simulink model "TosAeb"  (R2021a)
settings: Solver=FixedStepDiscrete  StartTime=0.0  StopTime=inf  FixedStep=0.01  RelTol=1e-3
undefined symbols (not defined in the file or in its folder): ACCModSts, AccSts1, AEB_ObjectMovingOrientation, AEB_ObjectMovingState, CN_BTCActive, CN_CurrentGearR, CN_CurrentGearR_DHT, CN_PTCActive, CN_VDCActive, CN_VSC_EspOff, CN_VSC_FCW_AEB_Error, CN_VSC_FCW_AEB_NoDispay, CN_VSC_FCW_AEB_PerfmDgdtn, CN_VSC_FCW_AEB_TempUnAvlbl, CN_VSC_VehStandstill, CNaccelAvoidMax, CNaccelAvoidMin, CNbrakePedalPress, CNcomfortStrLeftAccelMax, CNcomfortStrLeftAccelMin, CNcomfortStrRightAccelMax, CNcomfortStrRightAccelMin, CNDrvDoorOpen, CNDrvModeEco, CNDrvModeNorm, CNDrvModeOther, CNDrvModeSport, CNDrvSBRreminder, CNNull, CNobjInvalidID, CNPassDoorOpen, CNPassSBRreminder, CNPEBSAccelNegInf, CNPEBSAccelNull, CNPEBSTimeInf, CNPEBSTimeNull, CNSTOPMAX, CNSTOPNULL, CNsubjWidth, CNSwtOFF, CNSwtON, CNTTCMAX, CNTTCNULL, CNVehConfigAEBCycNone, CNVehConfigAEBNone, CNVehConfigAEBPedNone, CNVehConfigFCWNone, CNVehConfigJANone, CollisionSeverity, FALSE, FCWSnvty, JACycObjCalcInfoDefaultParams, JAPedObjCalcInfoDefaultParams, JAVehObjCalcInfoDefaultParams, latCycObjCalcInfoDefaultParams, latPedObjCalcInfoDefaultParams, longObjCalcInfoDefaultParams, ModSts, movingStatus, ObjectType ... (+1112)
libraries: simulink x3031, AEBSFunctionLib x50, Ta_Library x33, AEBSStateMachineLib x23, Mylibrary x12, simulink_extras x7, ObjSelect_lib x3, dspstat3 x2
(part 13 of 102)

== system TosAeb/AEB_Objsel/TA_Processor/AEB_ObjSelect/TargetSelect/Lat_ObjSelect/Lat_ValidNumber ==
  LatObj_Valid : Inport
  Add : Sum
  Compare To Constant : simulink/Logic and Bit Operations/Compare To Constant  relop===  const=1  OutDataTypeStr=boolean
  Compare To Constant1 : simulink/Logic and Bit Operations/Compare To Constant  relop=>  const=1  OutDataTypeStr=boolean
  Compare To Constant2 : simulink/Logic and Bit Operations/Compare To Constant  relop===  const=1  OutDataTypeStr=boolean
  Constant : Constant  Value=0  OutDataTypeStr=uint8
  Constant1 : Constant  OutDataTypeStr=uint8
  Constant2 : Constant  OutDataTypeStr=uint8
  For Iterator : ForIterator  IterationLimit=32
  Index Vector : MultiPortSwitch  Inputs=1
  Logical Operator : Logic  AllPortsSameDT=off  OutDataTypeStr=boolean
  Saturation1 : Saturate  UpperLimit=254  LowerLimit=0
  Switch : Switch  Criteria=u2 > Threshold
  Switch1 : Switch  Criteria=u2 > Threshold
  Switch2 : Switch  Criteria=u2 > Threshold
  Unit Delay : UnitDelay  SampleTime=-1  HasFrameUpgradeWarning=on
  Lat_ObjNum : Outport
  wires:
  LatObj_Valid/1 -> Index Vector/2
  For Iterator/1 -> [ID], Index Vector/1
  Index Vector/1 -> Compare To Constant/1
  Compare To Constant1/1 -> Switch/2
  Constant/1 -> Switch2/3
  Compare To Constant/1 -> Switch1/2, Logical Operator/1
  Add/1 -> Switch1/1
  Constant1/1 -> Add/2
  Unit Delay/1 -> Add/1, Switch1/3
  [ID] -> Compare To Constant1/1
  Switch1/1 -> Switch/1
  Switch/1 -> Saturation1/1
  Saturation1/1 -> Lat_ObjNum/1, Unit Delay/1
  Compare To Constant2/1 -> Logical Operator/2
  [ID] -> Compare To Constant2/1
  Logical Operator/1 -> Switch2/2
  Constant2/1 -> Switch2/1
  Switch2/1 -> Switch/3

== system TosAeb/AEB_Objsel/TA_Processor/AEB_ObjSelect/TargetSelect/Long_ObjSelect/Obj_IDConfirm ==
  TA_ObjInfo : Inport
  VehSelfData : Inport  Port=2
  Boudingbox_Calculate : Inport  Port=3
  Obj_Coef : Inport  Port=4
  TTI : Inport  Port=5
  AccTgtIdn : Inport  Port=6
  Overlap_Rate : Inport  Port=7
  Robust : Subsystem
  Target_Select_decle : Subsystem
  ValidTarget_ID : Outport
  ID_num : Outport  Port=2
  long_TTC : Outport  Port=3
  wires:
  Target_Select_decle/1(ValidTarget_ID) -> Robust/1(ValidTarget_ID)
  Target_Select_decle/2(Num) -> Robust/2(Num_IN)
  Target_Select_decle/3(long_TTC) -> long_TTC/1
  Overlap_Rate/1 -> Target_Select_decle/7(Overlap_Rate)
  AccTgtIdn/1 -> Target_Select_decle/6(AccTgtIdn)
  Boudingbox_Calculate/1 -> Target_Select_decle/3(Boudingbox_Calculate)
  TA_ObjInfo/1 -> Target_Select_decle/1(TA_ObjInfo)
  TTI/1 -> Target_Select_decle/5(TTI)
  Obj_Coef/1 -> Target_Select_decle/4(Obj_Coef)
  Robust/1(ID) -> ValidTarget_ID/1
  VehSelfData/1 -> Target_Select_decle/2(VehSelfData)
  Robust/2(Num) -> ID_num/1

== system TosAeb/AEB_Objsel/TA_Processor/PreProcessor/ObjectPrecessor/TAObjInfoCalc/For Each Subsystem ==
  Cam_ObsID_u8 : Inport
  Cam_ObsValid_u8 : Inport  Port=2
  Cam_ObsAge_u16 : Inport  Port=3
  Cam_ObsConfidence_u8 : Inport  Port=4
  Cam_ObsMotionCategory_u8 : Inport  Port=5
  Cam_ObsMotionStatus_u8 : Inport  Port=6
  Cam_ObsMeasureStatus_u8 : Inport  Port=7
  Cam_ObsClass_u8 : Inport  Port=8
  Cam_ObsVhSubType_u8 : Inport  Port=9
  Cam_ObsPedSubType_u8 : Inport  Port=10
  Cam_ObsPedPos_u8 : Inport  Port=11
  Cam_ObsPedOrientation_u8 : Inport  Port=12
  Cam_ObsLaneAssign_u8 : Inport  Port=13
  Cam_ObsLength_s : Inport  Port=14
  Cam_ObsWidth_s : Inport  Port=15
  Cam_ObsHeight_s : Inport  Port=16
  Cam_ObsCIPV_b : Inport  Port=17
  Cam_ObsMCP_b : Inport  Port=18
  Cam_ObsBlinker_u8 : Inport  Port=19
  Cam_ObsBrkLgt_b : Inport  Port=20
  Cam_ObsCenterAngle_s : Inport  Port=21
  Cam_ObsPosType_u8 : Inport  Port=22
  Cam_ObsPosX_s : Inport  Port=23
  Cam_ObsPosY_s : Inport  Port=24
  Cam_ObsVelX_s : Inport  Port=25
  Cam_ObsVelY_s : Inport  Port=26
  Cam_ObsAccX_s : Inport  Port=27
  Cam_ObsPosXSTD_s : Inport  Port=28
  Cam_ObsPosYSTD_s : Inport  Port=29
  Cam_ObsVelXSTD_s : Inport  Port=30
  Cam_ObsVelYSTD_s : Inport  Port=31
  Cam_ObsAccY_s : Inport  Port=32
  Cam_ObsAngle_s : Inport  Port=33
  Cam_ObsAngleSTD_s : Inport  Port=34
  Cam_ObsVelXAbs_s : Inport  Port=35
  Cam_ObsVelYAbs_s : Inport  Port=36
  Cam_ObsCornerPntX_s : Inport  Port=37
  Cam_ObsCutInDis_s : Inport  Port=38
  Cam_ObsCornerPntY_s : Inport  Port=39
  Cam_ObsCutInFlg_u8 : Inport  Port=40
  Cam_ObsCutInSpd_s : Inport  Port=41
  Cam_ObsCutInSpdValid_b : Inport  Port=42
  Cam_ObsProNotOnRoad_b : Inport  Port=43
  Cam_ObsProCoverdByOthers_b : Inport  Port=44
  Cam_ObsProObserveJump_b : Inport  Port=45
  Cam_ObsProObserveSide_b : Inport  Port=46
  Cam_ObsProBtTapLevel1_b : Inport  Port=47
  Cam_ObsProBtTapLevel2_b : Inport  Port=48
  Cam_ObsProBtTapLevel3_b : Inport  Port=49
  IDForEach : Inport  Port=50  Partition=on  PartitionDimension=1  PartitionWidth=1  PartitionOffset=0
  VehSelfIn : Inport  Port=51
  For Each : ForEach  DisableCoverage=on  ShowIterationIndex=on
  ForEachInput : Subsystem
  Info_Check1 : Subsystem
  Terminator : Terminator
  Fus_ObjID : Outport  ConcatenationDimension=1
  Fus_ObjOrientation : Outport  Port=2  ConcatenationDimension=1
  Fus_ObjState : Outport  Port=3  ConcatenationDimension=1
  Fus_ObjfusType : Outport  Port=4  ConcatenationDimension=1
  Fus_ObjLaneAssign : Outport  Port=5  ConcatenationDimension=1
  Fus_ObjDy : Outport  Port=6  ConcatenationDimension=1
  Fus_ObjDx : Outport  Port=7  ConcatenationDimension=1
  Fus_ObjVx : Outport  Port=8  ConcatenationDimension=1
  Fus_ObjVy : Outport  Port=9  ConcatenationDimension=1
  Fus_ObjQuality : Outport  Port=10  ConcatenationDimension=1
  Fus_ObjAy : Outport  Port=11  ConcatenationDimension=1
  Fus_ObjAx : Outport  Port=12  ConcatenationDimension=1
  Fus_ObjVxAbs : Outport  Port=13  ConcatenationDimension=1
  Fus_ObjVyAbs : Outport  Port=14  ConcatenationDimension=1
  Fus_ObjHeadAngle : Outport  Port=15  ConcatenationDimension=1
  Fus_ObjType : Outport  Port=16  ConcatenationDimension=1
  Fus_ObjLength : Outport  Port=17  ConcatenationDimension=1
  Fus_ObjWidth : Outport  Port=18  ConcatenationDimension=1
  Fus_ObjHight : Outport  Port=19  ConcatenationDimension=1
  Fus_dxSTD : Outport  Port=20  ConcatenationDimension=1
  Fus_dySTD : Outport  Port=21  ConcatenationDimension=1
  Fus_VelxSTD : Outport  Port=22  ConcatenationDimension=1
  Fus_VelySTD : Outport  Port=23  ConcatenationDimension=1
  Fus_HeadAngleSTD : Outport  Port=24  ConcatenationDimension=1
  Fus_CenterAngle : Outport  Port=25  ConcatenationDimension=1
  Fus_AxAbs : Outport  Port=26  ConcatenationDimension=1
  Fus_AyAbs : Outport  Port=27  ConcatenationDimension=1
  wires:
  VehSelfIn/1 -> Info_Check1/2(VehSelfIn)
  Info_Check1/1(Fus_ObjID) -> Fus_ObjID/1
  Info_Check1/2(Fus_ObjOrientation) -> Fus_ObjOrientation/1
  Info_Check1/3(Fus_ObjState) -> Fus_ObjState/1
  Info_Check1/4(Fus_ObjfusType) -> Fus_ObjfusType/1
  Info_Check1/5(Fus_ObjLaneAssign) -> Fus_ObjLaneAssign/1
  Info_Check1/6(Fus_ObjDy) -> Fus_ObjDy/1
  Info_Check1/7(Fus_ObjDx) -> Fus_ObjDx/1
  Info_Check1/8(Fus_ObjVx) -> Fus_ObjVx/1
  Info_Check1/9(Fus_ObjVy) -> Fus_ObjVy/1
  Info_Check1/10(Fus_ObjQuality) -> Fus_ObjQuality/1
  Info_Check1/11(Fus_ObjAy) -> Fus_ObjAy/1
  Info_Check1/12(Fus_ObjAx) -> Fus_ObjAx/1
  Info_Check1/13(Fus_ObjVxAbs) -> Fus_ObjVxAbs/1
  Info_Check1/14(Fus_ObjVyAbs) -> Fus_ObjVyAbs/1
  Info_Check1/15(Fus_ObjHeadAngle) -> Fus_ObjHeadAngle/1
  Info_Check1/16(Fus_ObjType) -> Fus_ObjType/1
  Info_Check1/17(Fus_ObjLength) -> Fus_ObjLength/1
  Info_Check1/18(Fus_ObjWidth) -> Fus_ObjWidth/1
  Info_Check1/19(Fus_ObjHight) -> Fus_ObjHight/1
  Info_Check1/20(Fus_dxSTD) -> Fus_dxSTD/1
  Info_Check1/21(Fus_dySTD) -> Fus_dySTD/1
  Info_Check1/22(Fus_VelxSTD) -> Fus_VelxSTD/1
  Info_Check1/23(Fus_VelySTD) -> Fus_VelySTD/1
  Info_Check1/24(Fus_HeadAngleSTD) -> Fus_HeadAngleSTD/1
  Info_Check1/25(Fus_CenterAngle) -> Fus_CenterAngle/1
  Info_Check1/26(Fus_AxAbs) -> Fus_AxAbs/1
  Info_Check1/27(Fus_AyAbs) -> Fus_AyAbs/1
  ForEachInput/1(Obs_Infomation) -> Info_Check1/1(Obs_Infomation)
  Cam_ObsID_u8/1 -> ForEachInput/2(Cam_ObsID_u8)
  Cam_ObsValid_u8/1 -> ForEachInput/3(Cam_ObsValid_u8)
  Cam_ObsAge_u16/1 -> ForEachInput/4(Cam_ObsAge_u16)
  Cam_ObsConfidence_u8/1 -> ForEachInput/5(Cam_ObsConfidence_u8)
  Cam_ObsMotionCategory_u8/1 -> ForEachInput/6(Cam_ObsMotionCategory_u8)
  Cam_ObsMotionStatus_u8/1 -> ForEachInput/7(Cam_ObsMotionStatus_u8)
  Cam_ObsMeasureStatus_u8/1 -> ForEachInput/8(Cam_ObsMeasureStatus_u8)
  Cam_ObsClass_u8/1 -> ForEachInput/9(Cam_ObsClass_u8)
  Cam_ObsVhSubType_u8/1 -> ForEachInput/10(Cam_ObsVhSubType_u8)
  Cam_ObsPedSubType_u8/1 -> ForEachInput/11(Cam_ObsPedSubType_u8)
  Cam_ObsPedPos_u8/1 -> ForEachInput/12(Cam_ObsPedPos_u8)
  Cam_ObsPedOrientation_u8/1 -> ForEachInput/13(Cam_ObsPedOrientation_u8)
  Cam_ObsLaneAssign_u8/1 -> ForEachInput/14(Cam_ObsLaneAssign_u8)
  Cam_ObsLength_s/1 -> ForEachInput/15(Cam_ObsLength_s)
  Cam_ObsWidth_s/1 -> ForEachInput/16(Cam_ObsWidth_s)
  Cam_ObsHeight_s/1 -> ForEachInput/17(Cam_ObsHeight_s)
  Cam_ObsCIPV_b/1 -> ForEachInput/18(Cam_ObsCIPV_b)
  Cam_ObsMCP_b/1 -> ForEachInput/19(Cam_ObsMCP_b)
  Cam_ObsBlinker_u8/1 -> ForEachInput/20(Cam_ObsBlinker_u8)
  Cam_ObsBrkLgt_b/1 -> ForEachInput/21(Cam_ObsBrkLgt_b)
  Cam_ObsCenterAngle_s/1 -> ForEachInput/22(Cam_ObsCenterAngle_s)
  Cam_ObsPosType_u8/1 -> ForEachInput/23(Cam_ObsPosType_u8)
  Cam_ObsPosX_s/1 -> ForEachInput/24(Cam_ObsPosX_s)
  Cam_ObsPosY_s/1 -> ForEachInput/25(Cam_ObsPosY_s)
  Cam_ObsVelX_s/1 -> ForEachInput/26(Cam_ObsVelX_s)
  Cam_ObsVelY_s/1 -> ForEachInput/27(Cam_ObsVelY_s)
  Cam_ObsAccX_s/1 -> ForEachInput/28(Cam_ObsAccX_s)
  Cam_ObsPosXSTD_s/1 -> ForEachInput/29(Cam_ObsPosXSTD_s)
  Cam_ObsPosYSTD_s/1 -> ForEachInput/30(Cam_ObsPosYSTD_s)
  Cam_ObsVelXSTD_s/1 -> ForEachInput/31(Cam_ObsVelXSTD_s)
  Cam_ObsVelYSTD_s/1 -> ForEachInput/32(Cam_ObsVelYSTD_s)
  Cam_ObsAccY_s/1 -> ForEachInput/33(Cam_ObsAccY_s)
  Cam_ObsAngle_s/1 -> ForEachInput/34(Cam_ObsAngle_s)
  Cam_ObsAngleSTD_s/1 -> ForEachInput/35(Cam_ObsAngleSTD_s)
  Cam_ObsVelXAbs_s/1 -> ForEachInput/36(Cam_ObsVelXAbs_s)
  Cam_ObsVelYAbs_s/1 -> ForEachInput/37(Cam_ObsVelYAbs_s)
  Cam_ObsCornerPntX_s/1 -> ForEachInput/38(Cam_ObsCornerPntX_s)
  Cam_ObsCutInDis_s/1 -> ForEachInput/39(Cam_ObsCutInDis_s)
  Cam_ObsCornerPntY_s/1 -> ForEachInput/40(Cam_ObsCornerPntY_s)
  Cam_ObsCutInFlg_u8/1 -> ForEachInput/41(Cam_ObsCutInFlg_u8)
  Cam_ObsCutInSpd_s/1 -> ForEachInput/42(Cam_ObsCutInSpd_s)
  Cam_ObsCutInSpdValid_b/1 -> ForEachInput/43(Cam_ObsCutInSpdValid_b)
  Cam_ObsProNotOnRoad_b/1 -> ForEachInput/44(Cam_ObsProNotOnRoad_b)
  Cam_ObsProCoverdByOthers_b/1 -> ForEachInput/45(Cam_ObsProCoverdByOthers_b)
  Cam_ObsProObserveJump_b/1 -> ForEachInput/46(Cam_ObsProObserveJump_b)
  Cam_ObsProObserveSide_b/1 -> ForEachInput/47(Cam_ObsProObserveSide_b)
  Cam_ObsProBtTapLevel1_b/1 -> ForEachInput/48(Cam_ObsProBtTapLevel1_b)
  Cam_ObsProBtTapLevel2_b/1 -> ForEachInput/49(Cam_ObsProBtTapLevel2_b)
  Cam_ObsProBtTapLevel3_b/1 -> ForEachInput/50(Cam_ObsProBtTapLevel3_b)
  IDForEach/1 -> Terminator/1
  For Each/1 -> ForEachInput/1(Index)

== system TosAeb/AEB_Objsel/TA_Processor/PreProcessor/ObjectPrecessor/TAObjInfoCalc/Signal_Assignment ==
  Fus_ObjID : Inport
  Fus_ObjOrientation : Inport  Port=2
  Fus_ObjState : Inport  Port=3
  Fus_ObjfusType : Inport  Port=4
  Fus_ObjLaneAssign : Inport  Port=5
  Fus_ObjDy : Inport  Port=6
  Fus_ObjDx : Inport  Port=7
  Fus_ObjVx : Inport  Port=8
  Fus_ObjVy : Inport  Port=9
  Fus_ObjQuality : Inport  Port=10
  Fus_ObjAy : Inport  Port=11
  Fus_ObjAx : Inport  Port=12
  Fus_ObjVxAbs : Inport  Port=13
  Fus_ObjVyAbs : Inport  Port=14
  Fus_ObjHeadAngle : Inport  Port=15
  Fus_ObjType : Inport  Port=16
  Fus_ObjLength : Inport  Port=17
  Fus_ObjWidth : Inport  Port=18
  Fus_ObjHight : Inport  Port=19
  Fus_dxSTD : Inport  Port=20
  Fus_dySTD : Inport  Port=21
  Fus_VelxSTD : Inport  Port=22
  Fus_VelySTD : Inport  Port=23
  Fus_HeadAngleSTD : Inport  Port=24
  Fus_CenterAngle : Inport  Port=25
  Fus_AxAbs : Inport  Port=26
  Fus_AyAbs : Inport  Port=27
  Bus Creator : BusCreator  Inputs=27  OutDataTypeStr=Bus: TA_ObjInfo  NonVirtualBus=on
  Signal Conversion{,1..26} (27 alike) : SignalConversion  OverrideOpt=off
  TA_ObjInfo : Outport
  wires:
  Fus_ObjID/1 -> Signal Conversion/1
  Fus_ObjState/1 -> Signal Conversion2/1
  Fus_ObjOrientation/1 -> Signal Conversion3/1
  Fus_ObjfusType/1 -> Signal Conversion4/1
  Fus_ObjType/1 -> Signal Conversion1/1
  Fus_ObjLaneAssign/1 -> Signal Conversion5/1
  Fus_ObjDx/1 -> Signal Conversion6/1
  Fus_ObjDy/1 -> Signal Conversion7/1
  Fus_dxSTD/1 -> Signal Conversion8/1
  Fus_dySTD/1 -> Signal Conversion9/1
  Fus_ObjVx/1 -> Signal Conversion10/1
  Fus_ObjVy/1 -> Signal Conversion11/1
  Fus_VelxSTD/1 -> Signal Conversion12/1
  Fus_VelySTD/1 -> Signal Conversion13/1
  Fus_ObjVxAbs/1 -> Signal Conversion14/1
  Fus_ObjVyAbs/1 -> Signal Conversion15/1
  Fus_ObjLength/1 -> Signal Conversion16/1
  Fus_ObjWidth/1 -> Signal Conversion17/1
  Fus_ObjHight/1 -> Signal Conversion18/1
  Fus_ObjAy/1 -> Signal Conversion20/1
  Fus_ObjAx/1 -> Signal Conversion19/1
  Fus_AxAbs/1 -> Signal Conversion21/1
  Fus_AyAbs/1 -> Signal Conversion22/1
  Fus_ObjQuality/1 -> Signal Conversion23/1
  Fus_ObjHeadAngle/1 -> Signal Conversion25/1
  Fus_HeadAngleSTD/1 -> Signal Conversion26/1
  Fus_CenterAngle/1 -> Signal Conversion24/1
  Signal Conversion21/1 -> Bus Creator/22  "TA_ObjAxAbs"
  Signal Conversion22/1 -> Bus Creator/23  "TA_ObjAyAbs"
  Signal Conversion20/1 -> Bus Creator/21  "TA_ObjAy"
  Signal Conversion23/1 -> Bus Creator/24  "TA_ObjConfidence"
  Signal Conversion19/1 -> Bus Creator/20  "TA_ObjAx"
  Signal Conversion24/1 -> Bus Creator/25  "TA_ObjCenterAngle"
  Signal Conversion18/1 -> Bus Creator/19  "TA_ObjHight"
  Signal Conversion17/1 -> Bus Creator/18  "TA_ObjWidth"
  Signal Conversion25/1 -> Bus Creator/26  "TA_ObjHeadAngle"
  Signal Conversion16/1 -> Bus Creator/17  "TA_ObjLength"
  Signal Conversion26/1 -> Bus Creator/27  "TA_ObjHeadAngleStd"
  Signal Conversion15/1 -> Bus Creator/16  "TA_ObjVyAbs"
  Signal Conversion14/1 -> Bus Creator/15  "TA_ObjVxAbs"
  Signal Conversion13/1 -> Bus Creator/14  "TA_ObjVyStd"
  Signal Conversion12/1 -> Bus Creator/13  "TA_ObjVxStd"
  Signal Conversion11/1 -> Bus Creator/12  "TA_ObjVy"
  Signal Conversion10/1 -> Bus Creator/11  "TA_ObjVx"
  Signal Conversion9/1 -> Bus Creator/10  "TA_ObjDyStd"
  Signal Conversion8/1 -> Bus Creator/9  "TA_ObjDxStd"
  Signal Conversion7/1 -> Bus Creator/8  "TA_ObjDy"
  Signal Conversion6/1 -> Bus Creator/7  "TA_ObjDx"
  Signal Conversion5/1 -> Bus Creator/6  "TA_ObjLaneAssign"
  Signal Conversion4/1 -> Bus Creator/5  "TA_ObjfusType"
  Signal Conversion3/1 -> Bus Creator/4  "TA_ObjOrientation"
  Signal Conversion2/1 -> Bus Creator/3  "TA_ObjState"
  Signal Conversion1/1 -> Bus Creator/2  "TA_ObjType"
  Signal Conversion/1 -> Bus Creator/1  "TA_ObjID"
  Bus Creator/1 -> TA_ObjInfo/1

== system TosAeb/AEB_Objsel/TA_Processor/PreProcessor/VehiclePreProcessor/CalculateCurvature/LowPassFilter ==
  note: Filter constant in low pass filter for pinion angle rate.
  note: 0.2
  PinionAngleRate_in : Inport
  Constant1 : Constant  Value=P_TA_PinionAgRateFilConInAsyEvlrCritEve
  LP1_1 : Subsystem
  PinionAngleRate : Outport
  wires:
  PinionAngleRate_in/1 -> LP1_1/1(U)
  Constant1/1 -> LP1_1/2(TsOverTau)
  LP1_1/1(Y) -> PinionAngleRate/1  "PinionAngleRate"

== system TosAeb/AEB_Objsel/TA_Processor/PreProcessor/VehiclePreProcessor/CalculateCurvature/SafeDivide ==
  Numerator : Inport  OutDataTypeStr=DataType
      settings: AttributesFormatString=DT=%<OutDataTypeStr>
  Denominator : Inport  Port=2  OutDataTypeStr=DataType
      settings: AttributesFormatString=DT=%<OutDataTypeStr>
  SafeDivide : MATLAB Function
  Quotient : Outport  OutDataTypeStr=DataType
      settings: AttributesFormatString=DT=%<OutDataTypeStr>
  wires:
  SafeDivide/1(Quotient) -> Quotient/1
  Denominator/1 -> SafeDivide/2(Denominator)  "Denominator"
  Numerator/1 -> SafeDivide/1(Numerator)  "Numerator"

== system TosAeb/AEB_Objsel/TA_Processor/PreProcessor/VehiclePreProcessor/CalculateCurvature/SafeDivide1 ==
  Numerator : Inport  OutDataTypeStr=DataType
      settings: AttributesFormatString=DT=%<OutDataTypeStr>
  Denominator : Inport  Port=2  OutDataTypeStr=DataType
      settings: AttributesFormatString=DT=%<OutDataTypeStr>
  SafeDivide : MATLAB Function
  Quotient : Outport  OutDataTypeStr=DataType
      settings: AttributesFormatString=DT=%<OutDataTypeStr>
  wires:
  SafeDivide/1(Quotient) -> Quotient/1
  Denominator/1 -> SafeDivide/2(Denominator)  "Denominator"
  Numerator/1 -> SafeDivide/1(Numerator)  "Numerator"

== system TosAeb/AEB_Objsel/TA_Processor/PreProcessor/VehiclePreProcessor/CalculateCurvature/StaticBicycleModelC0 ==
  note: 注：本模块使用方向盘角度作为输入
  VLgt : Inport
  PinionSteerAg : Inport  Port=2
  CalculateCurvatureCoG : Subsystem
  CalculateCurvatureRear : Subsystem
  CalculateDistanceToAxles : Subsystem
  CalculateSlip : Subsystem
  CalculateWheelAngle : Subsystem
  IntermediateCalculation : Subsystem
  CurvatureRear : Outport
  wires:
  CalculateCurvatureCoG/2(CurvatureCoG) -> CalculateSlip/1(CurvatureCoG)
  CalculateDistanceToAxles/1(DistCoGToRearAxle) -> [DistRe], CalculateCurvatureCoG/2(DistCoGToRearAxle)
  CalculateWheelAngle/1(WheelAngle) -> CalculateCurvatureCoG/1(WheelAngle)
  CalculateCurvatureCoG/1(RadiusCoG) -> CalculateCurvatureRear/1(RadiusCOG)
  VLgt/1 -> IntermediateCalculation/1(Speed)
  CalculateCurvatureRear/1(CurvatureRear) -> CurvatureRear/1
  PinionSteerAg/1 -> CalculateWheelAngle/1(PinionSteerAg)
  CalculateDistanceToAxles/2(DistCoGToFrontAxle) -> [DistFront], CalculateCurvatureCoG/3(DistCoGToFrontAxle)
  IntermediateCalculation/1(MassSpeedSquaredDivWheelBase) -> CalculateSlip/4(MassSpeedSquaredDivWheelBase), CalculateCurvatureCoG/4(MassSpeedSquaredDivWheelBase)
  [DistRe] -> CalculateSlip/2(DistCoGToRearAxle)
  [DistFront] -> CalculateSlip/3(DistCoGToFrontAxle)
  [DistRe] -> CalculateCurvatureRear/2(DistCoGToRearAxle)
  CalculateSlip/1(SlipAngle) -> CalculateCurvatureRear/3(SlipAngle)

== system TosAeb/AEB_Objsel/TA_Processor/PreProcessor/VehiclePreProcessor/CalculateCurvature/StaticBicycleModelC1 ==
  note: 注：本模块使用方向盘角速率作为输入
  VLgt : Inport
  PinionSteerAgRate : Inport  Port=2
  CalculateCurvatureCoG : Subsystem
  CalculateCurvatureRear : Subsystem
  CalculateDistanceToAxles : Subsystem
  CalculateSlip : Subsystem
  CalculateWheelAngle : Subsystem
  IntermediateCalculation : Subsystem
  CurvatureRear : Outport
  wires:
  CalculateWheelAngle/1(WheelAngle) -> CalculateCurvatureCoG/1(WheelAngle)
  CalculateCurvatureCoG/2(CurvatureCoG) -> CalculateSlip/1(CurvatureCoG)
  CalculateDistanceToAxles/1(DistCoGToRearAxle) -> [DistRe], CalculateCurvatureCoG/2(DistCoGToRearAxle)
  [DistRe] -> CalculateCurvatureRear/2(DistCoGToRearAxle)
  CalculateSlip/1(SlipAngle) -> CalculateCurvatureRear/3(SlipAngle)
  [DistFront] -> CalculateSlip/3(DistCoGToFrontAxle)
  VLgt/1 -> IntermediateCalculation/1(Speed)
  CalculateCurvatureRear/1(CurvatureRear) -> CurvatureRear/1
  CalculateCurvatureCoG/1(RadiusCoG) -> CalculateCurvatureRear/1(RadiusCOG)
  IntermediateCalculation/1(MassSpeedSquaredDivWheelBase) -> CalculateSlip/4(MassSpeedSquaredDivWheelBase), CalculateCurvatureCoG/4(MassSpeedSquaredDivWheelBase)
  [DistRe] -> CalculateSlip/2(DistCoGToRearAxle)
  CalculateDistanceToAxles/2(DistCoGToFrontAxle) -> [DistFront], CalculateCurvatureCoG/3(DistCoGToFrontAxle)
  PinionSteerAgRate/1 -> CalculateWheelAngle/1(PinionSteerAgRate)

== system TosAeb/AEB_Objsel/TA_Processor/PreProcessor/VehiclePreProcessor/CalculateCurvature/Weighting ==
  X1 : Inport
  WeightFactor : Inport  Port=2
  X2 : Inport  Port=3
  Add : Sum  Description=_3p3p4p5.ACC.000_11_  Inputs=-+
  Add1 : Sum  Description=_3p3p4p5.ACC.000_12_
  Constant : Constant  Description=_3p3p4p5.ACC.000_13_  Value=single(1)
  Product : Product  Description=_3p3p4p5.ACC.000_19_  RndMeth=Zero
  Product1 : Product  Description=_3p3p4p5.ACC.000_20_  RndMeth=Zero
  Saturation : Saturate  Description=_3p3p4p5.ACC.000_21_  UpperLimit=1  LowerLimit=0
  Y : Outport
  wires:
  WeightFactor/1 -> Saturation/1
  Saturation/1 -> Add/1, Product/2
  Add1/1 -> Y/1
  Product1/1 -> Add1/2
  Add/1 -> Product1/1
  X2/1 -> Product1/2
  Constant/1 -> Add/2
  Product/1 -> Add1/1
  X1/1 -> Product/1

== system TosAeb/AEB_Objsel/TA_Processor/PreProcessor/VehiclePreProcessor/CalculateCurvature/Weighting1 ==
  X1 : Inport
  WeightFactor : Inport  Port=2
  X2 : Inport  Port=3
  Add : Sum  Description=_3p3p4p5.ACC.000_11_  Inputs=-+
  Add1 : Sum  Description=_3p3p4p5.ACC.000_12_
  Constant : Constant  Description=_3p3p4p5.ACC.000_13_  Value=single(1)
  Product : Product  Description=_3p3p4p5.ACC.000_19_  RndMeth=Zero
  Product1 : Product  Description=_3p3p4p5.ACC.000_20_  RndMeth=Zero
  Saturation : Saturate  Description=_3p3p4p5.ACC.000_21_  UpperLimit=1  LowerLimit=0
  Y : Outport
  wires:
  WeightFactor/1 -> Saturation/1
  Saturation/1 -> Add/1, Product/2
  Add1/1 -> Y/1
  Product1/1 -> Add1/2
  Add/1 -> Product1/1
  X2/1 -> Product1/2
  Constant/1 -> Add/2
  Product/1 -> Add1/1
  X1/1 -> Product/1

== system TosAeb/AEB_Objsel/TA_Processor/PreProcessor/VehiclePreProcessor/PathEstimn_Calculate/For Iterator PahEstimn ==
  Curvature : Inport
  VehSelf : Inport  Port=2
  CurvatureRate : Inport  Port=3
  CheckVehicleStopping : Subsystem
  ComputeVehicleStateAtT1 : Subsystem
  For Iterator : ForIterator  IterationLimit=4
  InitialControlPointsCalculation : Subsystem
  PahEstimn1 : Subsystem
  SetVehicleStateAtT0 : Subsystem
  PahEstimn : Outport
  wires:
  For Iterator/1 -> [Index]
  [VehicleMotionT1] -> SetVehicleStateAtT0/5(VehicleMotionT1)  "VehicleMotionT1"
  [Index] -> SetVehicleStateAtT0/4(Index)  "Index"
  VehSelf/1 -> SetVehicleStateAtT0/1(VehSelf)
  SetVehicleStateAtT0/1(VehicleMotionT0) -> InitialControlPointsCalculation/2(VehicleMotionT0), CheckVehicleStopping/1(VehicleMotionT0), ComputeVehicleStateAtT1/1(VehicleMotionT0)  "VehicleMotionT0"
  CheckVehicleStopping/1(StoppingDistances) -> ComputeVehicleStateAtT1/2(StoppingDistances), InitialControlPointsCalculation/1(StoppingDistances)  "StoppingDistances"
  InitialControlPointsCalculation/1(ControlPoints) -> ComputeVehicleStateAtT1/3(ControlPoints), PahEstimn1/1(ControlPoints)
  ComputeVehicleStateAtT1/1(VehicleMotionT1) -> [VehicleMotionT1]
  [Index] -> PahEstimn1/2(Index)  "Index"
  PahEstimn1/1(PahEstimn) -> PahEstimn/1
  Curvature/1 -> SetVehicleStateAtT0/2(Curvature)
  CurvatureRate/1 -> SetVehicleStateAtT0/3(CurvatureRate)

== system TosAeb/ELK_Objsel/Target_Selection/If Action Subsystem/ELK_TargetSelection/If Action Threat_Assessment_Left/Delay_Trig ==
  Input : Inport
  Delay_Cnt : Inport  Port=2
  Chart : Stateflow chart  TreatAsAtomicUnit=on
  Output : Outport
  wires:
  Chart/1(Delay_Trig) -> Output/1
  Delay_Cnt/1 -> Chart/2(Delay_Trig_count)
  Input/1 -> Chart/1(Input)

== system TosAeb/ELK_Objsel/Target_Selection/If Action Subsystem/ELK_TargetSelection/If Action Threat_Assessment_Left/Delay_Trig1 ==
  Input : Inport
  Delay_Cnt : Inport  Port=2
  Chart : Stateflow chart  TreatAsAtomicUnit=on
  Output : Outport
  wires:
  Input/1 -> Chart/1(Input)
  Delay_Cnt/1 -> Chart/2(Delay_Trig_count)
  Chart/1(Delay_Trig) -> Output/1

== system TosAeb/ELK_Objsel/Target_Selection/If Action Subsystem/ELK_TargetSelection/If Action Threat_Assessment_Left/First_Order_Lag_Filter ==
  filt_loop_time_msec : Inport
  filt_tau_msec : Inport  Port=2
  unfiltered_input : Inport  Port=3
  Product2 : Product  Description=_GWMAB30P4.LKAFN.000_153_  Inputs=**  InputSameDT=on  OutDataTypeStr=Inherit: Same as first input
  Saturation : Saturate  Description=_GWMAB30P4.LKAFN.000_154_  UpperLimit=65535  LowerLimit=-65535
  Subsystem : Subsystem
  Sum : Sum  Description=_GWMAB30P4.LKAFN.000_155_  Inputs=+-  InputSameDT=on  OutDataTypeStr=Inherit: Same as first input
  Sum1 : Sum  Description=_GWMAB30P4.LKAFN.000_156_  InputSameDT=on  OutDataTypeStr=Inherit: Same as first input
  Unit Delay : UnitDelay  Description=_GWMAB30P4.LKAFN.000_157_  SampleTime=-1  HasFrameUpgradeWarning=on
  filtered_output : Outport
  wires:
  Subsystem/1(Gain) -> Product2/1
  filt_loop_time_msec/1 -> Subsystem/1(CL_TIME)
  Sum/1 -> Product2/2
  filt_tau_msec/1 -> Subsystem/2(time_constant)
  Sum1/1 -> Saturation/1
  unfiltered_input/1 -> Sum/1
  Product2/1 -> Sum1/1
  Saturation/1 -> filtered_output/1, Unit Delay/1
  Unit Delay/1 -> Sum/2, Sum1/2

== system TosAeb/ELK_Objsel/Target_Selection/If Action Subsystem/ELK_TargetSelection/If Action Threat_Assessment_Left/First_Order_Lag_Filter1 ==
  filt_loop_time_msec : Inport
  filt_tau_msec : Inport  Port=2
  unfiltered_input : Inport  Port=3
  Product2 : Product  Description=_GWMAB30P4.LKAFN.000_153_  Inputs=**  InputSameDT=on  OutDataTypeStr=Inherit: Same as first input
  Saturation : Saturate  Description=_GWMAB30P4.LKAFN.000_154_  UpperLimit=65535  LowerLimit=-65535
  Subsystem : Subsystem
  Sum : Sum  Description=_GWMAB30P4.LKAFN.000_155_  Inputs=+-  InputSameDT=on  OutDataTypeStr=Inherit: Same as first input
  Sum1 : Sum  Description=_GWMAB30P4.LKAFN.000_156_  InputSameDT=on  OutDataTypeStr=Inherit: Same as first input
  Unit Delay : UnitDelay  Description=_GWMAB30P4.LKAFN.000_157_  SampleTime=-1  HasFrameUpgradeWarning=on
  filtered_output : Outport
  wires:
  Unit Delay/1 -> Sum1/2, Sum/2
  Saturation/1 -> Unit Delay/1, filtered_output/1
  Product2/1 -> Sum1/1
  unfiltered_input/1 -> Sum/1
  Sum1/1 -> Saturation/1
  filt_tau_msec/1 -> Subsystem/2(time_constant)
  Sum/1 -> Product2/2
  filt_loop_time_msec/1 -> Subsystem/1(CL_TIME)
  Subsystem/1(Gain) -> Product2/1

== system TosAeb/ELK_Objsel/Target_Selection/If Action Subsystem/ELK_TargetSelection/If Action Threat_Assessment_Left/Trigger Hold ==
  Trigger : Inport
  hold_time : Inport  Port=2
  Chart : Stateflow chart  TreatAsAtomicUnit=on
  Out : Outport
  wires:
  Chart/1(Out) -> Out/1
  Trigger/1 -> Chart/1(Trigger)
  hold_time/1 -> Chart/2(hold_time)

== system TosAeb/ELK_Objsel/Target_Selection/If Action Subsystem/ELK_TargetSelection/If Action Threat_Assessment_Left/Trigger Hold1 ==
  Trigger : Inport
  hold_time : Inport  Port=2
  Chart : Stateflow chart  TreatAsAtomicUnit=on
  Out : Outport
  wires:
  hold_time/1 -> Chart/2(hold_time)
  Trigger/1 -> Chart/1(Trigger)
  Chart/1(Out) -> Out/1

== system TosAeb/ELK_Objsel/Target_Selection/If Action Subsystem/ELK_TargetSelection/If Action Threat_Assessment_Left1/Delay_Trig ==
  Input : Inport
  Delay_Cnt : Inport  Port=2
  Chart : Stateflow chart  TreatAsAtomicUnit=on
  Output : Outport
  wires:
  Input/1 -> Chart/1(Input)
  Delay_Cnt/1 -> Chart/2(Delay_Trig_count)
  Chart/1(Delay_Trig) -> Output/1

== system TosAeb/ELK_Objsel/Target_Selection/If Action Subsystem/ELK_TargetSelection/If Action Threat_Assessment_Left1/Delay_Trig1 ==
  Input : Inport
  Delay_Cnt : Inport  Port=2
  Chart : Stateflow chart  TreatAsAtomicUnit=on
  Output : Outport
  wires:
  Chart/1(Delay_Trig) -> Output/1
  Delay_Cnt/1 -> Chart/2(Delay_Trig_count)
  Input/1 -> Chart/1(Input)

== system TosAeb/ELK_Objsel/Target_Selection/If Action Subsystem/ELK_TargetSelection/If Action Threat_Assessment_Left1/First_Order_Lag_Filter ==
  filt_loop_time_msec : Inport
  filt_tau_msec : Inport  Port=2
  unfiltered_input : Inport  Port=3
  Product2 : Product  Description=_GWMAB30P4.LKAFN.000_153_  Inputs=**  InputSameDT=on  OutDataTypeStr=Inherit: Same as first input
  Saturation : Saturate  Description=_GWMAB30P4.LKAFN.000_154_  UpperLimit=65535  LowerLimit=-65535
  Subsystem : Subsystem
  Sum : Sum  Description=_GWMAB30P4.LKAFN.000_155_  Inputs=+-  InputSameDT=on  OutDataTypeStr=Inherit: Same as first input
  Sum1 : Sum  Description=_GWMAB30P4.LKAFN.000_156_  InputSameDT=on  OutDataTypeStr=Inherit: Same as first input
  Unit Delay : UnitDelay  Description=_GWMAB30P4.LKAFN.000_157_  SampleTime=-1  HasFrameUpgradeWarning=on
  filtered_output : Outport
  wires:
  Unit Delay/1 -> Sum1/2, Sum/2
  Saturation/1 -> Unit Delay/1, filtered_output/1
  Product2/1 -> Sum1/1
  unfiltered_input/1 -> Sum/1
  Sum1/1 -> Saturation/1
  filt_tau_msec/1 -> Subsystem/2(time_constant)
  Sum/1 -> Product2/2
  filt_loop_time_msec/1 -> Subsystem/1(CL_TIME)
  Subsystem/1(Gain) -> Product2/1

== system TosAeb/ELK_Objsel/Target_Selection/If Action Subsystem/ELK_TargetSelection/If Action Threat_Assessment_Left1/First_Order_Lag_Filter1 ==
  filt_loop_time_msec : Inport
  filt_tau_msec : Inport  Port=2
  unfiltered_input : Inport  Port=3
  Product2 : Product  Description=_GWMAB30P4.LKAFN.000_153_  Inputs=**  InputSameDT=on  OutDataTypeStr=Inherit: Same as first input
  Saturation : Saturate  Description=_GWMAB30P4.LKAFN.000_154_  UpperLimit=65535  LowerLimit=-65535
  Subsystem : Subsystem
  Sum : Sum  Description=_GWMAB30P4.LKAFN.000_155_  Inputs=+-  InputSameDT=on  OutDataTypeStr=Inherit: Same as first input
  Sum1 : Sum  Description=_GWMAB30P4.LKAFN.000_156_  InputSameDT=on  OutDataTypeStr=Inherit: Same as first input
  Unit Delay : UnitDelay  Description=_GWMAB30P4.LKAFN.000_157_  SampleTime=-1  HasFrameUpgradeWarning=on
  filtered_output : Outport
  wires:
  Subsystem/1(Gain) -> Product2/1
  filt_loop_time_msec/1 -> Subsystem/1(CL_TIME)
  Sum/1 -> Product2/2
  filt_tau_msec/1 -> Subsystem/2(time_constant)
  Sum1/1 -> Saturation/1
  unfiltered_input/1 -> Sum/1
  Product2/1 -> Sum1/1
  Saturation/1 -> filtered_output/1, Unit Delay/1
  Unit Delay/1 -> Sum/2, Sum1/2

== system TosAeb/ELK_Objsel/Target_Selection/If Action Subsystem/ELK_TargetSelection/If Action Threat_Assessment_Left1/Trigger Hold ==
  Trigger : Inport
  hold_time : Inport  Port=2
  Chart : Stateflow chart  TreatAsAtomicUnit=on
  Out : Outport
  wires:
  hold_time/1 -> Chart/2(hold_time)
  Trigger/1 -> Chart/1(Trigger)
  Chart/1(Out) -> Out/1

== system TosAeb/ELK_Objsel/Target_Selection/If Action Subsystem/ELK_TargetSelection/If Action Threat_Assessment_Left1/Trigger Hold1 ==
  Trigger : Inport
  hold_time : Inport  Port=2
  Chart : Stateflow chart  TreatAsAtomicUnit=on
  Out : Outport
  wires:
  Chart/1(Out) -> Out/1
  Trigger/1 -> Chart/1(Trigger)
  hold_time/1 -> Chart/2(hold_time)

== system TosAeb/ELK_Objsel/Target_Selection/If Action Subsystem/ELK_TargetSelection/If Action Threat_Assessment_Right/Delay_Trig ==
  Input : Inport
  Delay_Cnt : Inport  Port=2
  Chart : Stateflow chart  TreatAsAtomicUnit=on
  Output : Outport
  wires:
  Chart/1(Delay_Trig) -> Output/1
  Delay_Cnt/1 -> Chart/2(Delay_Trig_count)
  Input/1 -> Chart/1(Input)

== system TosAeb/ELK_Objsel/Target_Selection/If Action Subsystem/ELK_TargetSelection/If Action Threat_Assessment_Right/Delay_Trig1 ==
  Input : Inport
  Delay_Cnt : Inport  Port=2
  Chart : Stateflow chart  TreatAsAtomicUnit=on
  Output : Outport
  wires:
  Input/1 -> Chart/1(Input)
  Delay_Cnt/1 -> Chart/2(Delay_Trig_count)
  Chart/1(Delay_Trig) -> Output/1

== system TosAeb/ELK_Objsel/Target_Selection/If Action Subsystem/ELK_TargetSelection/If Action Threat_Assessment_Right/First_Order_Lag_Filter ==
  filt_loop_time_msec : Inport
  filt_tau_msec : Inport  Port=2
  unfiltered_input : Inport  Port=3
  Product2 : Product  Description=_GWMAB30P4.LKAFN.000_153_  Inputs=**  InputSameDT=on  OutDataTypeStr=Inherit: Same as first input
  Saturation : Saturate  Description=_GWMAB30P4.LKAFN.000_154_  UpperLimit=65535  LowerLimit=-65535
  Subsystem : Subsystem
  Sum : Sum  Description=_GWMAB30P4.LKAFN.000_155_  Inputs=+-  InputSameDT=on  OutDataTypeStr=Inherit: Same as first input
  Sum1 : Sum  Description=_GWMAB30P4.LKAFN.000_156_  InputSameDT=on  OutDataTypeStr=Inherit: Same as first input
  Unit Delay : UnitDelay  Description=_GWMAB30P4.LKAFN.000_157_  SampleTime=-1  HasFrameUpgradeWarning=on
  filtered_output : Outport
  wires:
  Subsystem/1(Gain) -> Product2/1
  filt_loop_time_msec/1 -> Subsystem/1(CL_TIME)
  Sum/1 -> Product2/2
  filt_tau_msec/1 -> Subsystem/2(time_constant)
  Sum1/1 -> Saturation/1
  unfiltered_input/1 -> Sum/1
  Product2/1 -> Sum1/1
  Saturation/1 -> filtered_output/1, Unit Delay/1
  Unit Delay/1 -> Sum/2, Sum1/2

== system TosAeb/ELK_Objsel/Target_Selection/If Action Subsystem/ELK_TargetSelection/If Action Threat_Assessment_Right/First_Order_Lag_Filter1 ==
  filt_loop_time_msec : Inport
  filt_tau_msec : Inport  Port=2
  unfiltered_input : Inport  Port=3
  Product2 : Product  Description=_GWMAB30P4.LKAFN.000_153_  Inputs=**  InputSameDT=on  OutDataTypeStr=Inherit: Same as first input
  Saturation : Saturate  Description=_GWMAB30P4.LKAFN.000_154_  UpperLimit=65535  LowerLimit=-65535
  Subsystem : Subsystem
  Sum : Sum  Description=_GWMAB30P4.LKAFN.000_155_  Inputs=+-  InputSameDT=on  OutDataTypeStr=Inherit: Same as first input
  Sum1 : Sum  Description=_GWMAB30P4.LKAFN.000_156_  InputSameDT=on  OutDataTypeStr=Inherit: Same as first input
  Unit Delay : UnitDelay  Description=_GWMAB30P4.LKAFN.000_157_  SampleTime=-1  HasFrameUpgradeWarning=on
  filtered_output : Outport
  wires:
  Unit Delay/1 -> Sum1/2, Sum/2
  Saturation/1 -> Unit Delay/1, filtered_output/1
  Product2/1 -> Sum1/1
  unfiltered_input/1 -> Sum/1
  Sum1/1 -> Saturation/1
  filt_tau_msec/1 -> Subsystem/2(time_constant)
  Sum/1 -> Product2/2
  filt_loop_time_msec/1 -> Subsystem/1(CL_TIME)
  Subsystem/1(Gain) -> Product2/1

== system TosAeb/ELK_Objsel/Target_Selection/If Action Subsystem/ELK_TargetSelection/If Action Threat_Assessment_Right/Trigger Hold ==
  Trigger : Inport
  hold_time : Inport  Port=2
  Chart : Stateflow chart  TreatAsAtomicUnit=on
  Out : Outport
  wires:
  Chart/1(Out) -> Out/1
  Trigger/1 -> Chart/1(Trigger)
  hold_time/1 -> Chart/2(hold_time)

== system TosAeb/ELK_Objsel/Target_Selection/If Action Subsystem/ELK_TargetSelection/If Action Threat_Assessment_Right/Trigger Hold1 ==
  Trigger : Inport
  hold_time : Inport  Port=2
  Chart : Stateflow chart  TreatAsAtomicUnit=on
  Out : Outport
  wires:
  hold_time/1 -> Chart/2(hold_time)
  Trigger/1 -> Chart/1(Trigger)
  Chart/1(Out) -> Out/1

== system TosAeb/ELK_Objsel/Target_Selection/If Action Subsystem/ELK_TargetSelection/If Action Threat_Assessment_Right1/Delay_Trig ==
  Input : Inport
  Delay_Cnt : Inport  Port=2
  Chart : Stateflow chart  TreatAsAtomicUnit=on
  Output : Outport
  wires:
  Input/1 -> Chart/1(Input)
  Delay_Cnt/1 -> Chart/2(Delay_Trig_count)
  Chart/1(Delay_Trig) -> Output/1

== system TosAeb/ELK_Objsel/Target_Selection/If Action Subsystem/ELK_TargetSelection/If Action Threat_Assessment_Right1/Delay_Trig1 ==
  Input : Inport
  Delay_Cnt : Inport  Port=2
  Chart : Stateflow chart  TreatAsAtomicUnit=on
  Output : Outport
  wires:
  Chart/1(Delay_Trig) -> Output/1
  Delay_Cnt/1 -> Chart/2(Delay_Trig_count)
  Input/1 -> Chart/1(Input)

== system TosAeb/ELK_Objsel/Target_Selection/If Action Subsystem/ELK_TargetSelection/If Action Threat_Assessment_Right1/First_Order_Lag_Filter ==
  filt_loop_time_msec : Inport
  filt_tau_msec : Inport  Port=2
  unfiltered_input : Inport  Port=3
  Product2 : Product  Description=_GWMAB30P4.LKAFN.000_153_  Inputs=**  InputSameDT=on  OutDataTypeStr=Inherit: Same as first input
  Saturation : Saturate  Description=_GWMAB30P4.LKAFN.000_154_  UpperLimit=65535  LowerLimit=-65535
  Subsystem : Subsystem
  Sum : Sum  Description=_GWMAB30P4.LKAFN.000_155_  Inputs=+-  InputSameDT=on  OutDataTypeStr=Inherit: Same as first input
  Sum1 : Sum  Description=_GWMAB30P4.LKAFN.000_156_  InputSameDT=on  OutDataTypeStr=Inherit: Same as first input
  Unit Delay : UnitDelay  Description=_GWMAB30P4.LKAFN.000_157_  SampleTime=-1  HasFrameUpgradeWarning=on
  filtered_output : Outport
  wires:
  Unit Delay/1 -> Sum1/2, Sum/2
  Saturation/1 -> Unit Delay/1, filtered_output/1
  Product2/1 -> Sum1/1
  unfiltered_input/1 -> Sum/1
  Sum1/1 -> Saturation/1
  filt_tau_msec/1 -> Subsystem/2(time_constant)
  Sum/1 -> Product2/2
  filt_loop_time_msec/1 -> Subsystem/1(CL_TIME)
  Subsystem/1(Gain) -> Product2/1

== system TosAeb/ELK_Objsel/Target_Selection/If Action Subsystem/ELK_TargetSelection/If Action Threat_Assessment_Right1/First_Order_Lag_Filter1 ==
  filt_loop_time_msec : Inport
  filt_tau_msec : Inport  Port=2
  unfiltered_input : Inport  Port=3
  Product2 : Product  Description=_GWMAB30P4.LKAFN.000_153_  Inputs=**  InputSameDT=on  OutDataTypeStr=Inherit: Same as first input
  Saturation : Saturate  Description=_GWMAB30P4.LKAFN.000_154_  UpperLimit=65535  LowerLimit=-65535
  Subsystem : Subsystem
  Sum : Sum  Description=_GWMAB30P4.LKAFN.000_155_  Inputs=+-  InputSameDT=on  OutDataTypeStr=Inherit: Same as first input
  Sum1 : Sum  Description=_GWMAB30P4.LKAFN.000_156_  InputSameDT=on  OutDataTypeStr=Inherit: Same as first input
  Unit Delay : UnitDelay  Description=_GWMAB30P4.LKAFN.000_157_  SampleTime=-1  HasFrameUpgradeWarning=on
  filtered_output : Outport
  wires:
  Subsystem/1(Gain) -> Product2/1
  filt_loop_time_msec/1 -> Subsystem/1(CL_TIME)
  Sum/1 -> Product2/2
  filt_tau_msec/1 -> Subsystem/2(time_constant)
  Sum1/1 -> Saturation/1
  unfiltered_input/1 -> Sum/1
  Product2/1 -> Sum1/1
  Saturation/1 -> filtered_output/1, Unit Delay/1
  Unit Delay/1 -> Sum/2, Sum1/2

== system TosAeb/ELK_Objsel/Target_Selection/If Action Subsystem/ELK_TargetSelection/If Action Threat_Assessment_Right1/Trigger Hold ==
  Trigger : Inport
  hold_time : Inport  Port=2
  Chart : Stateflow chart  TreatAsAtomicUnit=on
  Out : Outport
  wires:
  hold_time/1 -> Chart/2(hold_time)
  Trigger/1 -> Chart/1(Trigger)
  Chart/1(Out) -> Out/1

== system TosAeb/ELK_Objsel/Target_Selection/If Action Subsystem/ELK_TargetSelection/If Action Threat_Assessment_Right1/Trigger Hold1 ==
  Trigger : Inport
  hold_time : Inport  Port=2
  Chart : Stateflow chart  TreatAsAtomicUnit=on
  Out : Outport
  wires:
  Chart/1(Out) -> Out/1
  Trigger/1 -> Chart/1(Trigger)
  hold_time/1 -> Chart/2(hold_time)

== system TosAeb/ELK_Objsel/Target_Selection/If Action Subsystem/ELK_TargetSelection/Left/Subsystem ==
  a0 : Inport
  a1 : Inport  Port=2
  a2 : Inport  Port=3
  a3 : Inport  Port=4
  x : Inport  Port=5
  Add : Sum  Inputs=++++
  Math Function : Math  Operator=square  SignedPower=on  SaturateOnIntegerOverflow=on
  Product{,1..3} (4 alike) : Product
  Out1 : Outport
  wires:
  Product/1 -> Add/2
  Product1/1 -> Add/3
  Product2/1 -> Add/4
  Add/1 -> Out1/1
  a0/1 -> Add/1
  a1/1 -> Product/1
  x/1 -> Product3/2, Math Function/1, Product/2
  a2/1 -> Product1/1
  Math Function/1 -> Product3/1, Product1/2
  a3/1 -> Product2/1
  Product3/1 -> Product2/2

== system TosAeb/ELK_Objsel/Target_Selection/If Action Subsystem/ELK_TargetSelection/Left/Subsystem2 ==
  a1 : Inport
  a2 : Inport  Port=2
  a3 : Inport  Port=3
  x : Inport  Port=4
  Add : Sum  Inputs=+++
  Gain : Gain  Gain=2
  Gain1 : Gain  Gain=3
  Math Function : Math  Operator=square  SignedPower=on  SaturateOnIntegerOverflow=on
  Product1 : Product
  Product2 : Product
  Out1 : Outport
  wires:
  a1/1 -> Add/1
  Product1/1 -> Add/2
  Product2/1 -> Add/3
  Add/1 -> Out1/1
  x/1 -> Product1/2, Math Function/1
  a2/1 -> Gain/1
  a3/1 -> Gain1/1
  Math Function/1 -> Product2/2
  Gain/1 -> Product1/1
  Gain1/1 -> Product2/1

== system TosAeb/ELK_Objsel/Target_Selection/If Action Subsystem/ELK_TargetSelection/Right/Subsystem2 ==
  a0 : Inport
  a1 : Inport  Port=2
  a2 : Inport  Port=3
  a3 : Inport  Port=4
  x : Inport  Port=5
  Add : Sum  Inputs=++++
  Math Function : Math  Operator=square  SignedPower=on  SaturateOnIntegerOverflow=on
  Product{,1..3} (4 alike) : Product
  Out1 : Outport
  wires:
  Product3/1 -> Product2/2
  a3/1 -> Product2/1
  Math Function/1 -> Product1/2, Product3/1
  a2/1 -> Product1/1
  x/1 -> Product/2, Math Function/1, Product3/2
  a1/1 -> Product/1
  a0/1 -> Add/1
  Add/1 -> Out1/1
  Product2/1 -> Add/4
  Product1/1 -> Add/3
  Product/1 -> Add/2

== system TosAeb/ELK_Objsel/Target_Selection/If Action Subsystem/ELK_TargetSelection/Right/Subsystem3 ==
  a1 : Inport
  a2 : Inport  Port=2
  a3 : Inport  Port=3
  x : Inport  Port=4
  Add : Sum  Inputs=+++
  Gain : Gain  Gain=2
  Gain1 : Gain  Gain=3
  Math Function : Math  Operator=square  SignedPower=on  SaturateOnIntegerOverflow=on
  Product1 : Product
  Product2 : Product
  Out1 : Outport
  wires:
  a1/1 -> Add/1
  Product1/1 -> Add/2
  Product2/1 -> Add/3
  Add/1 -> Out1/1
  x/1 -> Product1/2, Math Function/1
  a2/1 -> Gain/1
  a3/1 -> Gain1/1
  Math Function/1 -> Product2/2
  Gain/1 -> Product1/1
  Gain1/1 -> Product2/1

== system TosAeb/FcwAebJa_Step_sys/ALG_AEBSystem/ALG_AEBController/AEB_FuncCondCalcApp/CycFunctionCondCalc/cycAEBfullSuppAborCond ==
  inputSignals : Inport
  cycAEBfullAborCondNoStandstillCalc : Subsystem
  cycAEBfullAborCondStandstillCalc : Subsystem
  cycAEBfullSuppCondCalc : Subsystem
  cycAEBfullSuppCond : Outport
  cycAEBfullAborCondNoStandstill : Outport  Port=2
  cycAEBfullAborCondStandstill : Outport  Port=3
  wires:
  inputSignals/1 -> cycAEBfullSuppCondCalc/1(inputSignals), cycAEBfullAborCondNoStandstillCalc/1(inputSignals), cycAEBfullAborCondStandstillCalc/1(inputSignals)
  cycAEBfullAborCondStandstillCalc/1(cycAEBfullAborCondStandstillFulfilled) -> cycAEBfullAborCondStandstill/1  "cycAEBfullAborCondStandstill"
  cycAEBfullAborCondNoStandstillCalc/1(cycAEBfullAborCondNoStandstillFulfilled) -> cycAEBfullAborCondNoStandstill/1  "cycAEBfullAborCondNoStandstill"
  cycAEBfullSuppCondCalc/1(cycAEBfullSuppCondFulfilled) -> cycAEBfullSuppCond/1  "cycAEBfullSuppCond"

== system TosAeb/FcwAebJa_Step_sys/ALG_AEBSystem/ALG_AEBController/AEB_FuncCondCalcApp/CycFunctionCondCalc/cycAEBpartSuppAborCond ==
  inputSignals : Inport
  cycAEBpartAborCondNoStandstillCalc : Subsystem
  cycAEBpartAborCondStandstillCalc : Subsystem
  cycAEBpartSuppCondCalc : Subsystem
  cycAEBpartSuppCond : Outport
  cycAEBpartAborCondNoStandstill : Outport  Port=2
  cycAEBpartAborCondStandstill : Outport  Port=3
  wires:
  cycAEBpartAborCondNoStandstillCalc/1(cycAEBpartAborCondNoStandstillFulfilled) -> cycAEBpartAborCondNoStandstill/1  "cycAEBpartAborCondNoStandstill"
  cycAEBpartAborCondStandstillCalc/1(cycAEBpartAborCondStandstillFulfilled) -> cycAEBpartAborCondStandstill/1  "cycAEBpartAborCondStandstill"
  inputSignals/1 -> cycAEBpartSuppCondCalc/1(inputSignals), cycAEBpartAborCondNoStandstillCalc/1(inputSignals), cycAEBpartAborCondStandstillCalc/1(inputSignals)
  cycAEBpartSuppCondCalc/1(cycAEBpartSuppCondFulfilled) -> cycAEBpartSuppCond/1  "cycAEBpartSuppCond"

== system TosAeb/FcwAebJa_Step_sys/ALG_AEBSystem/ALG_AEBController/AEB_FuncCondCalcApp/CycFunctionCondCalc/cycPrefillSuppAborCond ==
  inputSignals : Inport
  cycPrefillAborCondCalc : Subsystem
  cycPrefillSuppCondCalc : Subsystem
  cycPrefillSuppCond : Outport
  cycPrefillAborCond : Outport  Port=2
  wires:
  cycPrefillSuppCondCalc/1(cycPrefillSuppCondFulfilled) -> cycPrefillSuppCond/1  "cycPrefillSuppCond"
  cycPrefillAborCondCalc/1(cycPrefillAborCondFulfilled) -> cycPrefillAborCond/1  "cycPrefillAborCond"
  inputSignals/1 -> cycPrefillAborCondCalc/1(inputSignals), cycPrefillSuppCondCalc/1(inputSignals)

== system TosAeb/FcwAebJa_Step_sys/ALG_AEBSystem/ALG_AEBController/AEB_FuncCondCalcApp/JaCycFunctionCondCalc/JaCycAEBfullSuppAborCond ==
  inputSignals : Inport
  JaCycAEBfullAborCondCalc : Subsystem
  JaCycAEBfullSuppCondCalc : Subsystem
  JaCycAEBfullSuppCond : Outport
  JaCycAEBfullAborCondNoStandstill : Outport  Port=2
  JaCycAEBfullAborCondStandstill : Outport  Port=3
  wires:
  JaCycAEBfullAborCondCalc/2(JaCycAEBfullAborCondStandstillFulfilled) -> JaCycAEBfullAborCondStandstill/1
  inputSignals/1 -> JaCycAEBfullAborCondCalc/1(inputSignals), JaCycAEBfullSuppCondCalc/1(inputSignals)
  JaCycAEBfullAborCondCalc/1(JaCycAEBfullAborCondNoStandstillFulfilled) -> JaCycAEBfullAborCondNoStandstill/1
  JaCycAEBfullSuppCondCalc/1(JaCycAEBfullSuppCond) -> JaCycAEBfullSuppCond/1

== system TosAeb/FcwAebJa_Step_sys/ALG_AEBSystem/ALG_AEBController/AEB_FuncCondCalcApp/JaCycFunctionCondCalc/JaCycPrefillSuppAborCond ==
  inputSignals : Inport
  JaCycPrefillAborCondCalc : Subsystem
  JaCycPrefillSuppCondCalc : Subsystem
  JaCycPrefillSuppCond : Outport
  JaCycPrefillAborCond : Outport  Port=2
  wires:
  JaCycPrefillSuppCondCalc/1(JaCycPrefillSuppCond) -> JaCycPrefillSuppCond/1
  JaCycPrefillAborCondCalc/1(JaCycPrefillAborCond) -> JaCycPrefillAborCond/1
  inputSignals/1 -> JaCycPrefillSuppCondCalc/1(inputSignals), JaCycPrefillAborCondCalc/1(inputSignals)

== system TosAeb/FcwAebJa_Step_sys/ALG_AEBSystem/ALG_AEBController/AEB_FuncCondCalcApp/JaPedFunctionCondCalc/AEBJAPedAEBfullSuppAborCond ==
  inputSignals : Inport
  AEBJAPedAEBfullAborCondCalc : Subsystem
  AEBJAPedAEBfullSuppCondCalc : Subsystem
  AEBJAPedAEBfullSuppCond : Outport
  AEBJAPedAEBfullAborCondNoStandstill : Outport  Port=2
  AEBJAPedAEBfullAborCondStandstill : Outport  Port=3
  wires:
  AEBJAPedAEBfullSuppCondCalc/1(AEBJAPedAEBfullSuppCondFulfilled) -> AEBJAPedAEBfullSuppCond/1  "AEBJAPedAEBfullSuppCond"
  AEBJAPedAEBfullAborCondCalc/1(AEBJAPedAEBfullAborCondNoStandstillFulfilled) -> AEBJAPedAEBfullAborCondNoStandstill/1  "AEBJAPedAEBfullAborCondNoStandstill"
  inputSignals/1 -> AEBJAPedAEBfullSuppCondCalc/1(inputSignals), AEBJAPedAEBfullAborCondCalc/1(inputSignals)
  AEBJAPedAEBfullAborCondCalc/2(AEBJAPedAEBfullAborCondStandstillFulfilled) -> AEBJAPedAEBfullAborCondStandstill/1  "AEBJAPedAEBfullAborCondStandstill"

== system TosAeb/FcwAebJa_Step_sys/ALG_AEBSystem/ALG_AEBController/AEB_FuncCondCalcApp/JaPedFunctionCondCalc/AEBJAPedPrefillSuppAborCond ==
  inputSignals : Inport
  AEBJAPedPrefillAborCondCalc : Subsystem
  AEBJAPedPrefillSuppCondCalc : Subsystem
  AEBJAPedPrefillSuppCond : Outport
  AEBJAPedPrefillAborCond : Outport  Port=2
  wires:
  inputSignals/1 -> AEBJAPedPrefillAborCondCalc/1(inputSignals), AEBJAPedPrefillSuppCondCalc/1(inputSignals)
  AEBJAPedPrefillAborCondCalc/1(AEBJAPedPrefillAborCondFulfilled) -> AEBJAPedPrefillAborCond/1  "AEBJAPedPrefillAborCond"
  AEBJAPedPrefillSuppCondCalc/1(AEBJAPedPrefillSuppCondFulfilled) -> AEBJAPedPrefillSuppCond/1  "AEBJAPedPrefillSuppCond"

== system TosAeb/FcwAebJa_Step_sys/ALG_AEBSystem/ALG_AEBController/AEB_FuncCondCalcApp/JaVehFunctionCondCalc/AEBJAVehAEBfullSuppAborCond ==
  inputSignals : Inport
  AEBJAVehAEBfullAborCondCalc : Subsystem
  AEBJAVehAEBfullSuppCondCalc : Subsystem
  AEBJAVehAEBfullSuppCond : Outport
  AEBJAVehAEBfullAborCondNoStandstill : Outport  Port=2
  AEBJAVehAEBfullAborCondStandstill : Outport  Port=3
  wires:
  AEBJAVehAEBfullAborCondCalc/2(AEBJAVehAEBfullAborCondStandstillFulfilled) -> AEBJAVehAEBfullAborCondStandstill/1  "AEBJAVehAEBfullAborCondStandstill"
  inputSignals/1 -> AEBJAVehAEBfullAborCondCalc/1(inputSignals), AEBJAVehAEBfullSuppCondCalc/1(inputSignals)
  AEBJAVehAEBfullAborCondCalc/1(AEBJAVehAEBfullAborCondNoStandstillFulfilled) -> AEBJAVehAEBfullAborCondNoStandstill/1  "AEBJAVehAEBfullAborCondNoStandstill"
  AEBJAVehAEBfullSuppCondCalc/1(AEBJAVehAEBfullSuppCondFulfilled) -> AEBJAVehAEBfullSuppCond/1  "AEBJAVehAEBfullSuppCond"

== system TosAeb/FcwAebJa_Step_sys/ALG_AEBSystem/ALG_AEBController/AEB_FuncCondCalcApp/JaVehFunctionCondCalc/AEBJAVehPrefillSuppAborCond ==
  inputSignals : Inport
  AEBJAVehPrefillAborCondCalc : Subsystem
  AEBJAVehPrefillSuppCondCalc : Subsystem
  AEBJAVehPrefillSuppCond : Outport
  AEBJAVehPrefillAborCond : Outport  Port=2
  wires:
  AEBJAVehPrefillSuppCondCalc/1(AEBJAVehPrefillSuppCondFulfilled) -> AEBJAVehPrefillSuppCond/1  "AEBJAVehPrefillSuppCond"
  AEBJAVehPrefillAborCondCalc/1(AEBJAVehPrefillAborCondFulfilled) -> AEBJAVehPrefillAborCond/1  "AEBJAVehPrefillAborCond"
  inputSignals/1 -> AEBJAVehPrefillAborCondCalc/1(inputSignals), AEBJAVehPrefillSuppCondCalc/1(inputSignals)

== system TosAeb/FcwAebJa_Step_sys/ALG_AEBSystem/ALG_AEBController/AEB_FuncCondCalcApp/LongFunctionCondCalc/vehABASuppAborCondCalc ==
  inputSignals : Inport
  vehABAAborCondCalc : Subsystem
  vehABASuppCondCalc : Subsystem
  vehABASuppCond : Outport
  vehABAAborCond : Outport  Port=2
  wires:
  vehABAAborCondCalc/1(vehABAAborCondFulfilled) -> vehABAAborCond/1  "vehABAAborCond"
  vehABASuppCondCalc/1(vehABASuppCondFulfilled) -> vehABASuppCond/1  "vehABASuppCond"
  inputSignals/1 -> vehABASuppCondCalc/1(inputSignals), vehABAAborCondCalc/1(inputSignals)
